simulink model "CCMMotor"  (R2022b)
settings: Solver=FixedStepDiscrete  StartTime=0.0  StopTime=10.0  FixedStep=0.01  RelTol=1e-3
undefined symbols (not defined in the file or in its folder): DcChlType, HFAChlType, MtrRoutStaEnum, P_Std_ReturnType, VGAChlType
(part 3 of 4)

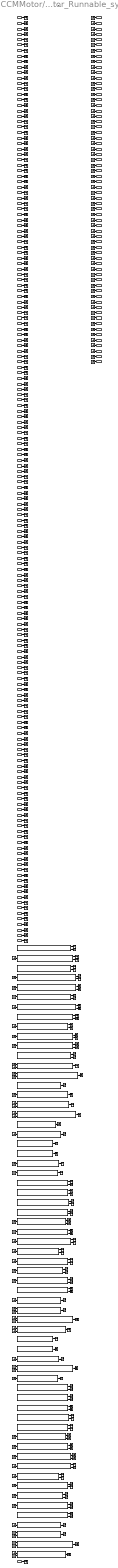
== system CCMMotor/CCMMotor_Runnable_sys ==
  ActrFrntReqForRowSecModDistbnRi_ActrFrntReqForRowSecModDistbnRi_read : Inport
  AirFlapPosnFrntReqForRowFirstForActrFlrLe_AirFlapPosnFrntReqForRowFirstForActrFlrLe_read : Inport  Port=2
  AirFlapPosnFrntReqForRowFirstForActrFlrRi_AirFlapPosnFrntReqForRowFirstForActrFlrRi_read : Inport  Port=3
  AirFlapPosnFrntReqForRowFirstForActrVentLe_AirFlapPosnFrntReqForRowFirstForActrVentLe_read : Inport  Port=4
  AirFlapPosnFrntReqForRowFirstForActrVentRi_AirFlapPosnFrntReqForRowFirstForActrVentRi_read : Inport  Port=5
  AirFlapPosnFrntReqForRowFirstForActrVentSideLe_AirFlapPosnFrntReqForRowFirstForActrVentSideLe_read : Inport  Port=6
  AirFlapPosnFrntReqForRowFirstForActrVentSideRi_AirFlapPosnFrntReqForRowFirstForActrVentSideRi_read : Inport  Port=7
  AirFlapPosnFrntReqForRowFirstForOsa_AirFlapPosnFrntReqForRowFirstForOsa_read : Inport  Port=8
  AirFlapPosnFrntReqForRowFirstForRecirc_AirFlapPosnFrntReqForRowFirstForRecirc_read : Inport  Port=9
  AirFlapPosnFrntReqForRowSecForActrTRi_AirFlapPosnFrntReqForRowSecForActrTRi_read : Inport  Port=10
  PwrMdAllMtrSlp_PwrMdAllMtrSlp_read : Inport  Port=11
  SysServCarCfgSts_SysServCarID_read : Inport  Port=12
  SysServCarCfgSts_SysServCCPAQSSts_read : Inport  Port=13
  SysServCarCfgSts_SysServCCPHEXSensorSts_read : Inport  Port=14
  SysServCarCfgSts_SysServCCPLin2AFUSts_read : Inport  Port=15
  SysServCarCfgSts_SysServCCPLin2AIUSts_read : Inport  Port=16
  SysServCarCfgSts_SysServCCPLin2CDSSts_read : Inport  Port=17
  SysServCarCfgSts_SysServCCPLin2PMSISts_read : Inport  Port=18
  SysServCarCfgSts_SysServCCPLin3VGASts_read : Inport  Port=19
  SysServCarCfgSts_SysServDUAirVentSnrSts_read : Inport  Port=20
  SysServCarCfgSts_SysServDUBlowerPwmSignSts_read : Inport  Port=21
  SysServCarCfgSts_SysServDUBlowerPwmSts_read : Inport  Port=22
  SysServCarCfgSts_SysServDUDCDefrostSts_read : Inport  Port=23
  SysServCarCfgSts_SysServDUDCModeSts_read : Inport  Port=24
  SysServCarCfgSts_SysServDUDCRecSts_read : Inport  Port=25
  SysServCarCfgSts_SysServDUDCSModeSts_read : Inport  Port=26
  SysServCarCfgSts_SysServDUDCSTLSts_read : Inport  Port=27
  SysServCarCfgSts_SysServDUDCTLSts_read : Inport  Port=28
  SysServCarCfgSts_SysServDUDCTRSts_read : Inport  Port=29
  SysServCarCfgSts_SysServDUFLFootSts_read : Inport  Port=30
  SysServCarCfgSts_SysServDUFLVentSts_read : Inport  Port=31
  SysServCarCfgSts_SysServDUFRFootSts_read : Inport  Port=32
  SysServCarCfgSts_SysServDUFRVentSts_read : Inport  Port=33
  SysServCarCfgSts_SysServDUIncarMotorSts_read : Inport  Port=34
  SysServCarCfgSts_SysServDULin1HBMFSts_read : Inport  Port=35
  SysServCarCfgSts_SysServDULin4HFAFSts_read : Inport  Port=36
  SysServCarCfgSts_SysServDUSFLFootSts_read : Inport  Port=37
  SysServCarCfgSts_SysServDUSFLVentSts_read : Inport  Port=38
  SysServCarCfgSts_SysServDUSFRFootSts_read : Inport  Port=39
  SysServCarCfgSts_SysServDUSFRVentSts_read : Inport  Port=40
  SysServCarCfgSts_SysServDUSnsrForFCmptmtTSts_read : Inport  Port=41
  SysServCarCfgSts_SysServDUSnsrForRair_read : Inport  Port=42
  SysServCarCfgSts_SysServDUSnsrForRCmptmtTSts_read : Inport  Port=43
  VehModMngtGlbSafe1_VehModMngtGlbSafe1_read : Inport  Port=44
  ReqForActrCal_ReqForActrCal_read : Inport  Port=45
  ActrFrntReqForRowFirstLeModDistbn_ActrFrntReqForRowFirstLeModDistbn_read : Inport  Port=46
  ActrFrntReqForRowFirstLeModDistbn_ActrFrntReqForRowFirstLeModDistbn_IsUpdated_read : Inport  Port=47
  ActrFrntReqForRowFirstModDistbn_ActrFrntReqForRowFirstModDistbn_read : Inport  Port=48
  ActrFrntReqForRowFirstModDistbn_ActrFrntReqForRowFirstModDistbn_IsUpdated_read : Inport  Port=49
  ActrFrntReqForRowSecModDistbnLe_ActrFrntReqForRowSecModDistbnLe_read : Inport  Port=50
  ActrFrntReqForRowSecModDistbnLe_ActrFrntReqForRowSecModDistbnLe_IsUpdated_read : Inport  Port=51
  ActrPosnFrnReqForOsaAndRecirc_ActrPosnFrnReqForOsaAndRecirc_read : Inport  Port=52
  ActrPosnFrnReqForOsaAndRecirc_ActrPosnFrnReqForOsaAndRecirc_IsUpdated_read : Inport  Port=53
  ActrReqForModDistbnRe_ActrReqForModDistbnRe_read : Inport  Port=54
  ActrReqForModDistbnRe_ActrReqForModDistbnRe_IsUpdated_read : Inport  Port=55
  AirFlapPosnFrntReqForRowFirstForActrDefrst_AirFlapPosnFrntReqForRowFirstForActrDefrst_read : Inport  Port=56
  AirFlapPosnFrntReqForRowFirstForActrDefrst_AirFlapPosnFrntReqForRowFirstForActrDefrst_IsUpdated_read : Inport  Port=57
  AirFlapPosnFrntReqForRowFirstForActrTLe_AirFlapPosnFrntReqForRowFirstForActrTLe_read : Inport  Port=58
  AirFlapPosnFrntReqForRowFirstForActrTLe_AirFlapPosnFrntReqForRowFirstForActrTLe_IsUpdated_read : Inport  Port=59
  AirFlapPosnFrntReqForRowFirstForActrTRi_AirFlapPosnFrntReqForRowFirstForActrTRi_read : Inport  Port=60
  AirFlapPosnFrntReqForRowFirstForActrTRi_AirFlapPosnFrntReqForRowFirstForActrTRi_IsUpdated_read : Inport  Port=61
  AirFlapPosnFrntReqForRowSecForActrTLe_AirFlapPosnFrntReqForRowSecForActrTLe_read : Inport  Port=62
  AirFlapPosnFrntReqForRowSecForActrTLe_AirFlapPosnFrntReqForRowSecForActrTLe_IsUpdated_read : Inport  Port=63
  CarCfgForClimaCtrl_CarCfgForAirClng_read : Inport  Port=64
  CarCfgForClimaCtrl_CarCfgForAirClnrAdv_read : Inport  Port=65
  CarCfgForClimaCtrl_CarCfgForAirFrag_read : Inport  Port=66
  CarCfgForClimaCtrl_CarCfgForAirMtrlSnsr_read : Inport  Port=67
  CarCfgForClimaCtrl_CarCfgForAirQly_read : Inport  Port=68
  CarCfgForClimaCtrl_CarCfgForAmntOfSeat_read : Inport  Port=69
  CarCfgForClimaCtrl_CarCfgForAutDemst_read : Inport  Port=70
  CarCfgForClimaCtrl_CarCfgForAuxWtrPmp_read : Inport  Port=71
  CarCfgForClimaCtrl_CarCfgForBRAND_read : Inport  Port=72
  CarCfgForClimaCtrl_CarCfgForBypVlvInHvac_read : Inport  Port=73
  CarCfgForClimaCtrl_CarCfgForClsVlvInHvac_read : Inport  Port=74
  CarCfgForClimaCtrl_CarCfgForCmprAcTyp_read : Inport  Port=75
  CarCfgForClimaCtrl_CarCfgForCmptmtClng_read : Inport  Port=76
  CarCfgForClimaCtrl_CarCfgForElectricLiftingModule_read : Inport  Port=77
  CarCfgForClimaCtrl_CarCfgForElecVentAdjmt_read : Inport  Port=78
  CarCfgForClimaCtrl_CarCfgForExtVentnClimaCtrl_read : Inport  Port=79
  CarCfgForClimaCtrl_CarCfgForFlcTyp_read : Inport  Port=80
  CarCfgForClimaCtrl_CarCfgForFoldCSD_read : Inport  Port=81
  CarCfgForClimaCtrl_CarCfgForFuTyp_read : Inport  Port=82
  CarCfgForClimaCtrl_CarCfgForGlassTyp_read : Inport  Port=83
  CarCfgForClimaCtrl_CarCfgForHeadRadar_read : Inport  Port=84
  CarCfgForClimaCtrl_CarCfgForHeatPmp_read : Inport  Port=85
  CarCfgForClimaCtrl_CarCfgForHeatrDurgDrvg_read : Inport  Port=86
  CarCfgForClimaCtrl_CarCfgForHeatrTyp_read : Inport  Port=87
  CarCfgForClimaCtrl_CarCfgForHvacFilTyp_read : Inport  Port=88
  CarCfgForClimaCtrl_CarCfgForHvacFlrSnsrForT_read : Inport  Port=89
  CarCfgForClimaCtrl_CarCfgForHvacHeatrSnsrT_read : Inport  Port=90
  CarCfgForClimaCtrl_CarCfgForHvacRe_read : Inport  Port=91
  CarCfgForClimaCtrl_CarCfgForHvacTyp_read : Inport  Port=92
  CarCfgForClimaCtrl_CarCfgForInteriorViewCamera_read : Inport  Port=93
  CarCfgForClimaCtrl_CarCfgForLifeDetection_read : Inport  Port=94
  CarCfgForClimaCtrl_CarCfgForLoVoltElecCfg_read : Inport  Port=95
  CarCfgForClimaCtrl_CarCfgForNavSysandIntnmap_read : Inport  Port=96
  CarCfgForClimaCtrl_CarCfgForNrOfDoor_read : Inport  Port=97
  CarCfgForClimaCtrl_CarCfgForPipeInFuTank_read : Inport  Port=98
  CarCfgForClimaCtrl_CarCfgForPowerSeats_read : Inport  Port=99
  CarCfgForClimaCtrl_CarCfgForPreClng_read : Inport  Port=100
  CarCfgForClimaCtrl_CarCfgForPrkgCDSNotAvl_read : Inport  Port=101
  CarCfgForClimaCtrl_CarCfgForPrkgClima_read : Inport  Port=102
  CarCfgForClimaCtrl_CarCfgForPrpsnTyp_read : Inport  Port=103
  CarCfgForClimaCtrl_CarCfgForPTCInFrontHVAC_read : Inport  Port=104
  CarCfgForClimaCtrl_CarCfgForRemEngStrtPreCdn_read : Inport  Port=105
  CarCfgForClimaCtrl_CarCfgForRoofHatch_read : Inport  Port=106
  CarCfgForClimaCtrl_CarCfgForSeatHeatgCal_read : Inport  Port=107
  CarCfgForClimaCtrl_CarCfgForSeatHeatgForRowFirst_read : Inport  Port=108
  CarCfgForClimaCtrl_CarCfgForSeatHeatgForRowSec_read : Inport  Port=109
  CarCfgForClimaCtrl_CarCfgForSeatVentnForRowFirst_read : Inport  Port=110
  CarCfgForClimaCtrl_CarCfgForSeatVentnForRowSec_read : Inport  Port=111
  CarCfgForClimaCtrl_CarCfgForSecRowVentnCenter_read : Inport  Port=112
  CarCfgForClimaCtrl_CarCfgForSecRowVentnSide_read : Inport  Port=113
  CarCfgForClimaCtrl_CarCfgForSmallFlowCyc_read : Inport  Port=114
  CarCfgForClimaCtrl_CarCfgForSmkSnsr_read : Inport  Port=115
  CarCfgForClimaCtrl_CarCfgForSnsrForCmptmtT_read : Inport  Port=116
  CarCfgForClimaCtrl_CarCfgForSnsrHumPrsnt_read : Inport  Port=117
  CarCfgForClimaCtrl_CarCfgForStartDevice_read : Inport  Port=118
  CarCfgForClimaCtrl_CarCfgForSteerWhlHeatg_read : Inport  Port=119
  CarCfgForClimaCtrl_CarCfgForSteerWhlPosn_read : Inport  Port=120
  CarCfgForClimaCtrl_CarCfgForSunSnsr_read : Inport  Port=121
  CarCfgForClimaCtrl_CarCfgForTelmNodTyp_read : Inport  Port=122
  CarCfgForClimaCtrl_CarCfgForTEMPEREDSTORAGEBOX_read : Inport  Port=123
  CarCfgForClimaCtrl_CarCfgForThermMngt_read : Inport  Port=124
  CarCfgForClimaCtrl_CarCfgForVehTyp_read : Inport  Port=125
  CarCfgForClimaCtrl_CarCfgForVentLiftMotorExiSts_read : Inport  Port=126
  CarCfgForClimaCtrl_CarCfgForVgaMotorExistSts_read : Inport  Port=127
  CarCfgForClimaCtrl_CarCfgForWadingAssistSystem_read : Inport  Port=128
  CarCfgForClimaCtrl_CarCfgForWindHeatd_read : Inport  Port=129
  CarCfgForClimaCtrl_CarCfgWindFollowHumanMov_read : Inport  Port=130
  SysServCarCfgSts_SysServCCPBGMNum_read : Inport  Port=131
  SysServCarCfgSts_SysServCCPLin2AQSSts_read : Inport  Port=132
  SysServCarCfgSts_SysServDUBlowerPwmSignTypeSts_read : Inport  Port=133
  SysServCarCfgSts_SysServDUDCFLMdeSts_read : Inport  Port=134
  SysServCarCfgSts_SysServDULin4HFAFStsH_read : Inport  Port=135
  SysServCarCfgSts_SysServDUReBlowerPwmSignSts_read : Inport  Port=136
  SysServCarCfgSts_SysServDUReBlowerPwmSts_read : Inport  Port=137
  SysServCarCfgSts_SysServFrntHEXSnsrPosn_read : Inport  Port=138
  SysServCarCfgSts_SysServLowSideRETXVSts_read : Inport  Port=139
  SysServCarCfgSts_SysServReHEXSnsrPosn_read : Inport  Port=140
  SysServCarCfgSts_SysServRHFASts_read : Inport  Port=141
  SysServCarCfgSts_SysServSnsrForFCmptmtTType_read : Inport  Port=142
  VentLiftModCaliReq_VentLiftModCaliReq_read : Inport  Port=143
  VentnDrvrLeXPsonReq_VentnDrvrLeXPsonReq_read : Inport  Port=144
  VentnDrvrLeXPsonReq_VentnDrvrLeXPsonReq_IsUpdated_read : Inport  Port=145
  VentnDrvrLeYPsonReq_VentnDrvrLeYPsonReq_read : Inport  Port=146
  VentnDrvrLeYPsonReq_VentnDrvrLeYPsonReq_IsUpdated_read : Inport  Port=147
  VentnDrvrRiXPsonReq_VentnDrvrRiXPsonReq_read : Inport  Port=148
  VentnDrvrRiXPsonReq_VentnDrvrRiXPsonReq_IsUpdated_read : Inport  Port=149
  VentnDrvrRiYPsonReq_VentnDrvrRiYPsonReq_read : Inport  Port=150
  VentnDrvrRiYPsonReq_VentnDrvrRiYPsonReq_IsUpdated_read : Inport  Port=151
  VentnLiftModPosnReq_VentnLiftModPosnReq_read : Inport  Port=152
  VentnLiftModPosnReq_VentnLiftModPosnReq_IsUpdated_read : Inport  Port=153
  VentnPassLeXPsonReq_VentnPassLeXPsonReq_read : Inport  Port=154
  VentnPassLeXPsonReq_VentnPassLeXPsonReq_IsUpdated_read : Inport  Port=155
  VentnPassLeYPsonReq_VentnPassLeYPsonReq_read : Inport  Port=156
  VentnPassLeYPsonReq_VentnPassLeYPsonReq_IsUpdated_read : Inport  Port=157
  VentnPassRiXPsonReq_VentnPassRiXPsonReq_read : Inport  Port=158
  VentnPassRiXPsonReq_VentnPassRiXPsonReq_IsUpdated_read : Inport  Port=159
  VentnPassRiYPsonReq_VentnPassRiYPsonReq_read : Inport  Port=160
  VentnPassRiYPsonReq_VentnPassRiYPsonReq_IsUpdated_read : Inport  Port=161
  VentnSecLeXPosnReq_VentnSecLeXPosnReq_read : Inport  Port=162
  VentnSecLeXPosnReq_VentnSecLeXPosnReq_IsUpdated_read : Inport  Port=163
  VentnSecLeYPosnReq_VentnSecLeYPosnReq_read : Inport  Port=164
  VentnSecLeYPosnReq_VentnSecLeYPosnReq_IsUpdated_read : Inport  Port=165
  VentnSecRiXPosnReq_VentnSecRiXPosnReq_read : Inport  Port=166
  VentnSecRiXPosnReq_VentnSecRiXPosnReq_IsUpdated_read : Inport  Port=167
  VentnSecRiYPosnReq_VentnSecRiYPosnReq_read : Inport  Port=168
  VentnSecRiYPosnReq_VentnSecRiYPosnReq_IsUpdated_read : Inport  Port=169
  WtrBafePsonReq_WtrBafePsonReq_read : Inport  Port=170
  BswLifeCycle_LifeCycle_read : Inport  Port=171
  function : TriggerPort  TriggerType=function-call  VariantControl=(inherit)  SampleTimeType=periodic  SampleTime=-1
  DcMotorParaService_DcMotorAllowSleepSts : FunctionCaller  FunctionPrototype=[Status,ERR] = DcMotorParaService_DcMotorAllowSleepSts()  OutputArgumentSpecifications=uint8(1), P_Std_ReturnType
  DcMotorParaService_GetMotorActualPositon : FunctionCaller  FunctionPrototype=[Postion,ERR] = DcMotorParaService_GetMotorActualPositon(Channel)  InputArgumentSpecifications=DcChlType(0)  OutputArgumentSpecifications=uint16(1), P_Std_ReturnType
  DcMotorParaService_GetMotorCailLearnSts : FunctionCaller  FunctionPrototype=[Status,ERR] = DcMotorParaService_GetMotorCailLearnSts()  OutputArgumentSpecifications=uint8(1), P_Std_ReturnType
  DcMotorParaService_GetMotorCalibrationStatus : FunctionCaller  FunctionPrototype=[Status,ERR] = DcMotorParaService_GetMotorCalibrationStatus(Channel)  InputArgumentSpecifications=DcChlType(0)  OutputArgumentSpecifications=uint8(1), P_Std_ReturnType
  DcMotorParaService_GetMotorDidWorkingStatus : FunctionCaller  FunctionPrototype=[Status,ERR] = DcMotorParaService_GetMotorDidWorkingStatus(Channel)  InputArgumentSpecifications=DcChlType(0)  OutputArgumentSpecifications=uint8(1), P_Std_ReturnType
  DcMotorParaService_GetMotorEndPosition : FunctionCaller  FunctionPrototype=[Postion,ERR] = DcMotorParaService_GetMotorEndPosition(Channel)  InputArgumentSpecifications=DcChlType(0)  OutputArgumentSpecifications=uint16(1), P_Std_ReturnType
  DcMotorParaService_GetMotorRequestPercent : FunctionCaller  FunctionPrototype=[Position,ERR] = DcMotorParaService_GetMotorRequestPercent(Channel)  InputArgumentSpecifications=DcChlType(0)  OutputArgumentSpecifications=uint16(1), P_Std_ReturnType
  DcMotorParaService_GetMotorRoutineCailSts : FunctionCaller  FunctionPrototype=[Status,ERR] = DcMotorParaService_GetMotorRoutineCailSts()  OutputArgumentSpecifications=uint8(1), P_Std_ReturnType
  DcMotorParaService_GetMotorRunTime : FunctionCaller  FunctionPrototype=[Runtime,ERR] = DcMotorParaService_GetMotorRunTime(Channel)  InputArgumentSpecifications=DcChlType(0)  OutputArgumentSpecifications=uint32(1), P_Std_ReturnType
  DcMotorParaService_GetMotorWorkingStatus : FunctionCaller  FunctionPrototype=[Status,ERR] = DcMotorParaService_GetMotorWorkingStatus(Channel)  InputArgumentSpecifications=DcChlType(0)  OutputArgumentSpecifications=uint8(1), P_Std_ReturnType
  DcMotorParaService_GetMotorZeroPosition : FunctionCaller  FunctionPrototype=[Position,ERR] = DcMotorParaService_GetMotorZeroPosition(Channel)  InputArgumentSpecifications=DcChlType(0)  OutputArgumentSpecifications=uint16(1), P_Std_ReturnType
  DcMotorParaService_GetRamAirErrorStatus : FunctionCaller  FunctionPrototype=[Status,ERR] = DcMotorParaService_GetRamAirErrorStatus()  OutputArgumentSpecifications=uint8(1), P_Std_ReturnType
  DcMotorParaService_SetMotorEndPosition : FunctionCaller  FunctionPrototype=ERR = DcMotorParaService_SetMotorEndPosition(Channel,Postion)  InputArgumentSpecifications=DcChlType(0), uint16(1)  OutputArgumentSpecifications=P_Std_ReturnType
  DcMotorParaService_SetMotorRequestPercent : FunctionCaller  FunctionPrototype=ERR = DcMotorParaService_SetMotorRequestPercent(Channel,Position)  InputArgumentSpecifications=DcChlType(0), uint16(1)  OutputArgumentSpecifications=P_Std_ReturnType
  DcMotorParaService_SetMotorRoutineCail : FunctionCaller  FunctionPrototype=ERR = DcMotorParaService_SetMotorRoutineCail()  OutputArgumentSpecifications=P_Std_ReturnType
  DcMotorParaService_SetMotorRoutineCailSts : FunctionCaller  FunctionPrototype=ERR = DcMotorParaService_SetMotorRoutineCailSts(Status)  InputArgumentSpecifications=uint8(1)  OutputArgumentSpecifications=P_Std_ReturnType
  DcMotorParaService_SetMotorRunTime : FunctionCaller  FunctionPrototype=ERR = DcMotorParaService_SetMotorRunTime(Channel,Runtime)  InputArgumentSpecifications=DcChlType(0), uint32(1)  OutputArgumentSpecifications=P_Std_ReturnType
  DcMotorParaService_SetMotorZeroPosition : FunctionCaller  FunctionPrototype=ERR = DcMotorParaService_SetMotorZeroPosition(Channel,Position)  InputArgumentSpecifications=DcChlType(0), uint16(1)  OutputArgumentSpecifications=P_Std_ReturnType
  DcMtrCaliServive_SetAllCalibrate : FunctionCaller  FunctionPrototype=ERR = DcMtrCaliServive_SetAllCalibrate()  OutputArgumentSpecifications=P_Std_ReturnType
  DcMtrCaliServive_SetMotorCalibrate : FunctionCaller  FunctionPrototype=ERR = DcMtrCaliServive_SetMotorCalibrate(Channel)  InputArgumentSpecifications=uint8(1)  OutputArgumentSpecifications=P_Std_ReturnType
  Ground_{1..117} (117 alike) : Ground
  HfaCaliService_SetAllCalibrate : FunctionCaller  FunctionPrototype=ERR = HfaCaliService_SetAllCalibrate()  OutputArgumentSpecifications=P_Std_ReturnType
  HfaCaliService_SetAllParaInit : FunctionCaller  FunctionPrototype=ERR = HfaCaliService_SetAllParaInit()  OutputArgumentSpecifications=P_Std_ReturnType
  HfaCaliService_SetMotorCalibrate : FunctionCaller  FunctionPrototype=ERR = HfaCaliService_SetMotorCalibrate(Channel)  InputArgumentSpecifications=HFAChlType(0)  OutputArgumentSpecifications=P_Std_ReturnType
  HfaCaliService_SetMotorParaInit : FunctionCaller  FunctionPrototype=ERR = HfaCaliService_SetMotorParaInit(Channel)  InputArgumentSpecifications=HFAChlType(0)  OutputArgumentSpecifications=P_Std_ReturnType
  HfaParaService_GetAllMotorCaliStatus : FunctionCaller  FunctionPrototype=[Status,ERR] = HfaParaService_GetAllMotorCaliStatus()  OutputArgumentSpecifications=uint8(1), P_Std_ReturnType
  HfaParaService_GetCailRoutineStatus : FunctionCaller  FunctionPrototype=[Status,ERR] = HfaParaService_GetCailRoutineStatus()  OutputArgumentSpecifications=MtrRoutStaEnum(0), P_Std_ReturnType
  HfaParaService_GetFreshRecErrorStatus : FunctionCaller  FunctionPrototype=[Status,ERR] = HfaParaService_GetFreshRecErrorStatus()  OutputArgumentSpecifications=uint8(1), P_Std_ReturnType
  HfaParaService_GetMotorAllowSleepSts : FunctionCaller  FunctionPrototype=[Status,ERR] = HfaParaService_GetMotorAllowSleepSts()  OutputArgumentSpecifications=uint8(1), P_Std_ReturnType
  HfaParaService_GetMotorCaliStatus : FunctionCaller  FunctionPrototype=[Status,ERR] = HfaParaService_GetMotorCaliStatus(Channel)  InputArgumentSpecifications=HFAChlType(0)  OutputArgumentSpecifications=uint8(1), P_Std_ReturnType
  HfaParaService_GetMotorCurrentPosition : FunctionCaller  FunctionPrototype=[Pos,ERR] = HfaParaService_GetMotorCurrentPosition(Channel)  InputArgumentSpecifications=HFAChlType(0)  OutputArgumentSpecifications=uint16(1), P_Std_ReturnType
  HfaParaService_GetMotorDidWorkingStatus : FunctionCaller  FunctionPrototype=[Status,ERR] = HfaParaService_GetMotorDidWorkingStatus(Channel)  InputArgumentSpecifications=HFAChlType(0)  OutputArgumentSpecifications=uint8(1), P_Std_ReturnType
  HfaParaService_GetMotorMoveCnt : FunctionCaller  FunctionPrototype=[Cnt,ERR] = HfaParaService_GetMotorMoveCnt(Channel)  InputArgumentSpecifications=HFAChlType(0)  OutputArgumentSpecifications=uint32(1), P_Std_ReturnType
  HfaParaService_GetMotorReqPosition : FunctionCaller  FunctionPrototype=[Position,ERR] = HfaParaService_GetMotorReqPosition(Channel)  InputArgumentSpecifications=HFAChlType(0)  OutputArgumentSpecifications=uint16(1), P_Std_ReturnType
  HfaParaService_GetMotorStepRange : FunctionCaller  FunctionPrototype=[Range,ERR] = HfaParaService_GetMotorStepRange(Channel)  InputArgumentSpecifications=HFAChlType(0)  OutputArgumentSpecifications=uint16(1), P_Std_ReturnType
  HfaParaService_GetMotorWorkingStatus : FunctionCaller  FunctionPrototype=[Status,ERR] = HfaParaService_GetMotorWorkingStatus(Channel)  InputArgumentSpecifications=HFAChlType(0)  OutputArgumentSpecifications=uint8(1), P_Std_ReturnType
  HfaParaService_GetRamAirErrorStatus : FunctionCaller  FunctionPrototype=[Status,ERR] = HfaParaService_GetRamAirErrorStatus()  OutputArgumentSpecifications=uint8(1), P_Std_ReturnType
  HfaParaService_SetCailRoutineStatus : FunctionCaller  FunctionPrototype=ERR = HfaParaService_SetCailRoutineStatus(Status)  InputArgumentSpecifications=MtrRoutStaEnum(0)  OutputArgumentSpecifications=P_Std_ReturnType
  HfaParaService_SetMotorMoveCnt : FunctionCaller  FunctionPrototype=ERR = HfaParaService_SetMotorMoveCnt(Channel,Cnt)  InputArgumentSpecifications=HFAChlType(0), uint32(1)  OutputArgumentSpecifications=P_Std_ReturnType
  HfaParaService_SetMotorRequestPosition : FunctionCaller  FunctionPrototype=ERR = HfaParaService_SetMotorRequestPosition(Channel,Present)  InputArgumentSpecifications=HFAChlType(0), uint16(1)  OutputArgumentSpecifications=P_Std_ReturnType
  HfaParaService_SetMotorStepRange : FunctionCaller  FunctionPrototype=ERR = HfaParaService_SetMotorStepRange(Channel,Range)  InputArgumentSpecifications=HFAChlType(0), uint16(1)  OutputArgumentSpecifications=P_Std_ReturnType
  Terminator_{1..272} (272 alike) : Terminator
  VgaCaliServive_SetAllCalibrate : FunctionCaller  FunctionPrototype=ERR = VgaCaliServive_SetAllCalibrate()  OutputArgumentSpecifications=P_Std_ReturnType
  VgaCaliServive_SetAllParaInit : FunctionCaller  FunctionPrototype=ERR = VgaCaliServive_SetAllParaInit()  OutputArgumentSpecifications=P_Std_ReturnType
  VgaCaliServive_SetMotorCalibrate : FunctionCaller  FunctionPrototype=ERR = VgaCaliServive_SetMotorCalibrate(Channel)  InputArgumentSpecifications=VGAChlType(0)  OutputArgumentSpecifications=P_Std_ReturnType
  VgaCaliServive_SetMotorCalibrateNew : FunctionCaller  FunctionPrototype=ERR = VgaCaliServive_SetMotorCalibrateNew(Channel,LearnType)  InputArgumentSpecifications=VGAChlType(0), uint8(1)  OutputArgumentSpecifications=P_Std_ReturnType
  VgaCaliServive_SetMotorParaInit : FunctionCaller  FunctionPrototype=ERR = VgaCaliServive_SetMotorParaInit(Channel)  InputArgumentSpecifications=VGAChlType(0)  OutputArgumentSpecifications=P_Std_ReturnType
  VgaParaService_GetAllMotorCaliStatus : FunctionCaller  FunctionPrototype=[Status,ERR] = VgaParaService_GetAllMotorCaliStatus()  OutputArgumentSpecifications=uint8(1), P_Std_ReturnType
  VgaParaService_GetCailRoutineStatus : FunctionCaller  FunctionPrototype=[Status,ERR] = VgaParaService_GetCailRoutineStatus()  OutputArgumentSpecifications=MtrRoutStaEnum(0), P_Std_ReturnType
  VgaParaService_GetFreshRecErrorStatus : FunctionCaller  FunctionPrototype=[Status,ERR] = VgaParaService_GetFreshRecErrorStatus()  OutputArgumentSpecifications=uint8(1), P_Std_ReturnType
  VgaParaService_GetFrntVgaErrorStatus : FunctionCaller  FunctionPrototype=[Status,ERR] = VgaParaService_GetFrntVgaErrorStatus()  OutputArgumentSpecifications=uint8(1), P_Std_ReturnType
  VgaParaService_GetMotorAllowSleepSts : FunctionCaller  FunctionPrototype=[Status,ERR] = VgaParaService_GetMotorAllowSleepSts()  OutputArgumentSpecifications=uint8(1), P_Std_ReturnType
  VgaParaService_GetMotorCaliStatus : FunctionCaller  FunctionPrototype=[Status,ERR] = VgaParaService_GetMotorCaliStatus(Channel)  InputArgumentSpecifications=VGAChlType(0)  OutputArgumentSpecifications=uint8(1), P_Std_ReturnType
  VgaParaService_GetMotorCurrentPosition : FunctionCaller  FunctionPrototype=[Pos,ERR] = VgaParaService_GetMotorCurrentPosition(Channel)  InputArgumentSpecifications=VGAChlType(0)  OutputArgumentSpecifications=uint8(1), P_Std_ReturnType
  VgaParaService_GetMotorCurrentPositionNew : FunctionCaller  FunctionPrototype=[Pos,ERR] = VgaParaService_GetMotorCurrentPositionNew(Channel)  InputArgumentSpecifications=VGAChlType(0)  OutputArgumentSpecifications=uint16(1), P_Std_ReturnType
  VgaParaService_GetMotorDidWorkingStatus : FunctionCaller  FunctionPrototype=[Status,ERR] = VgaParaService_GetMotorDidWorkingStatus(Channel)  InputArgumentSpecifications=VGAChlType(0)  OutputArgumentSpecifications=uint8(1), P_Std_ReturnType
  VgaParaService_GetMotorMoveCnt : FunctionCaller  FunctionPrototype=[Cnt,ERR] = VgaParaService_GetMotorMoveCnt(Channel)  InputArgumentSpecifications=VGAChlType(0)  OutputArgumentSpecifications=uint32(1), P_Std_ReturnType
  VgaParaService_GetMotorReqPosition : FunctionCaller  FunctionPrototype=[Position,ERR] = VgaParaService_GetMotorReqPosition(Channel)  InputArgumentSpecifications=VGAChlType(0)  OutputArgumentSpecifications=uint8(1), P_Std_ReturnType
  VgaParaService_GetMotorStepRange : FunctionCaller  FunctionPrototype=[Range,ERR] = VgaParaService_GetMotorStepRange(Channel)  InputArgumentSpecifications=VGAChlType(0)  OutputArgumentSpecifications=uint16(1), P_Std_ReturnType
  VgaParaService_GetMotorWorkingStatus : FunctionCaller  FunctionPrototype=[Status,ERR] = VgaParaService_GetMotorWorkingStatus(Channel)  InputArgumentSpecifications=VGAChlType(0)  OutputArgumentSpecifications=uint8(1), P_Std_ReturnType
  VgaParaService_GetRearVgaErrorStatus : FunctionCaller  FunctionPrototype=[Status,ERR] = VgaParaService_GetRearVgaErrorStatus()  OutputArgumentSpecifications=uint8(1), P_Std_ReturnType
  VgaParaService_SetCailRoutineStatus : FunctionCaller  FunctionPrototype=ERR = VgaParaService_SetCailRoutineStatus(Status)  InputArgumentSpecifications=MtrRoutStaEnum(0)  OutputArgumentSpecifications=P_Std_ReturnType
  VgaParaService_SetMotorMoveCnt : FunctionCaller  FunctionPrototype=ERR = VgaParaService_SetMotorMoveCnt(Channel,Cnt)  InputArgumentSpecifications=VGAChlType(0), uint32(1)  OutputArgumentSpecifications=P_Std_ReturnType
  VgaParaService_SetMotorRequestPosition : FunctionCaller  FunctionPrototype=ERR = VgaParaService_SetMotorRequestPosition(Channel,Present)  InputArgumentSpecifications=VGAChlType(0), uint16(1)  OutputArgumentSpecifications=P_Std_ReturnType
  VgaParaService_SetMotorStepRange : FunctionCaller  FunctionPrototype=ERR = VgaParaService_SetMotorStepRange(Channel,Range)  InputArgumentSpecifications=VGAChlType(0), uint16(1)  OutputArgumentSpecifications=P_Std_ReturnType
  ActrPosnActForModDistbnRe_ActrPosnActForModDistbnRe_write : Outport
      settings: EnsureOutportIsVirtual=on
  ActrPosnFrntActForRowFirstModDistbnLe_ActrPosnFrntActForRowFirstModDistbnLe_write : Outport  Port=2
      settings: EnsureOutportIsVirtual=on
  AirFlapFrntStsForRowFirstModDistbnLe_AirFlapFrntStsForRowFirstModDistbnLe_write : Outport  Port=3
      settings: EnsureOutportIsVirtual=on
  AirFlapStsForActModDistbnRe_AirFlapStsForActModDistbnRe_write : Outport  Port=4
      settings: EnsureOutportIsVirtual=on
  DriverVolThresholdLimitOfMotor_DriverVolThresholdLimitOfMotor_write : Outport  Port=5
      settings: EnsureOutportIsVirtual=on
  HvacOsaAndRecActrQfForHp_HvacOsaAndRecActrQfForHp_write : Outport  Port=6
      settings: EnsureOutportIsVirtual=on
  VentnLiftModPosnRsp_VentnLiftModPosnRsp_write : Outport  Port=7
      settings: EnsureOutportIsVirtual=on
  VentnLiftModStsRsp_VentnLiftModStsRsp_write : Outport  Port=8
      settings: EnsureOutportIsVirtual=on
  ActrFrntStsForRowFirstModDistbn_ActrFrntStsForRowFirstModDistbn_write : Outport  Port=9
      settings: EnsureOutportIsVirtual=on
  ActrFrntStsForRowSecModDistbnLe_ActrFrntStsForRowSecModDistbnLe_write : Outport  Port=10
      settings: EnsureOutportIsVirtual=on
  ActrFrntStsForRowSecModDistbnRi_ActrFrntStsForRowSecModDistbnRi_write : Outport  Port=11
      settings: EnsureOutportIsVirtual=on
  ActrPosnFrntActForOsaAndRecirc_ActrPosnFrntActForOsaAndRecirc_write : Outport  Port=12
      settings: EnsureOutportIsVirtual=on
  ActrPosnFrntActForRowFirstModDistbn_ActrPosnFrntActForRowFirstModDistbn_write : Outport  Port=13
      settings: EnsureOutportIsVirtual=on
  ActrPosnFrntActForRowSecModDistbnLe_ActrPosnFrntActForRowSecModDistbnLe_write : Outport  Port=14
      settings: EnsureOutportIsVirtual=on
  ActrPosnFrntActForRowSecModDistbnRi_ActrPosnFrntActForRowSecModDistbnRi_write : Outport  Port=15
      settings: EnsureOutportIsVirtual=on
  AirFlapFrntStsForRowFirstForActrDefrst_AirFlapFrntStsForRowFirstForActrDefrst_write : Outport  Port=16
      settings: EnsureOutportIsVirtual=on
  AirFlapFrntStsForRowFirstForActrFlrLe_AirFlapFrntStsForRowFirstForActrFlrLe_write : Outport  Port=17
      settings: EnsureOutportIsVirtual=on
  AirFlapFrntStsForRowFirstForActrFlrRi_AirFlapFrntStsForRowFirstForActrFlrRi_write : Outport  Port=18
      settings: EnsureOutportIsVirtual=on
  AirFlapFrntStsForRowFirstForActrTLe_AirFlapFrntStsForRowFirstForActrTLe_write : Outport  Port=19
      settings: EnsureOutportIsVirtual=on
  AirFlapFrntStsForRowFirstForActrTRi_AirFlapFrntStsForRowFirstForActrTRi_write : Outport  Port=20
      settings: EnsureOutportIsVirtual=on
  AirFlapFrntStsForRowFirstForActrVentLe_AirFlapFrntStsForRowFirstForActrVentLe_write : Outport  Port=21
      settings: EnsureOutportIsVirtual=on
  AirFlapFrntStsForRowFirstForActrVentRi_AirFlapFrntStsForRowFirstForActrVentRi_write : Outport  Port=22
      settings: EnsureOutportIsVirtual=on
  AirFlapFrntStsForRowFirstForActrVentSideLe_AirFlapFrntStsForRowFirstForActrVentSideLe_write : Outport  Port=23
      settings: EnsureOutportIsVirtual=on
  AirFlapFrntStsForRowFirstForActrVentSideRi_AirFlapFrntStsForRowFirstForActrVentSideRi_write : Outport  Port=24
      settings: EnsureOutportIsVirtual=on
  AirFlapFrntStsForRowFirstForOsa_AirFlapFrntStsForRowFirstForOsa_write : Outport  Port=25
      settings: EnsureOutportIsVirtual=on
  AirFlapFrntStsForRowFirstForOsaAndRecirc_AirFlapFrntStsForRowFirstForOsaAndRecirc_write : Outport  Port=26
      settings: EnsureOutportIsVirtual=on
  AirFlapFrntStsForRowFirstForRecirc_AirFlapFrntStsForRowFirstForRecirc_write : Outport  Port=27
      settings: EnsureOutportIsVirtual=on
  AirFlapFrntStsForRowSecForActrTLe_AirFlapFrntStsForRowSecForActrTLe_write : Outport  Port=28
      settings: EnsureOutportIsVirtual=on
  AirFlapFrntStsForRowSecForActrTRi_AirFlapFrntStsForRowSecForActrTRi_write : Outport  Port=29
      settings: EnsureOutportIsVirtual=on
  AirFlapPosnFrntActForRowFirstForActrDefrst_AirFlapPosnFrntActForRowFirstForActrDefrst_write : Outport  Port=30
      settings: EnsureOutportIsVirtual=on
  AirFlapPosnFrntActForRowFirstForActrFlrLe_AirFlapPosnFrntActForRowFirstForActrFlrLe_write : Outport  Port=31
      settings: EnsureOutportIsVirtual=on
  AirFlapPosnFrntActForRowFirstForActrFlrRi_AirFlapPosnFrntActForRowFirstForActrFlrRi_write : Outport  Port=32
      settings: EnsureOutportIsVirtual=on
  AirFlapPosnFrntActForRowFirstForActrTLe_AirFlapPosnFrntActForRowFirstForActrTLe_write : Outport  Port=33
      settings: EnsureOutportIsVirtual=on
  AirFlapPosnFrntActForRowFirstForActrTRi_AirFlapPosnFrntActForRowFirstForActrTRi_write : Outport  Port=34